simulink model "CCMMotor"  (R2022b)
settings: Solver=FixedStepDiscrete  StartTime=0.0  StopTime=10.0  FixedStep=0.01  RelTol=1e-3
undefined symbols (not defined in the file or in its folder): DcChlType, HFAChlType, MtrRoutStaEnum, P_Std_ReturnType, VGAChlType
(part 1 of 4)
settings Model: Creator=blue.ge  LastModifiedBy=blue.ge
settings DataIOCC: SaveFormat=StructureWithTime
settings OptimizationCC: DefaultParameterBehavior=Inlined  EnhancedBackFolding=on  PassReuseOutputArgsAs=Individual arguments  ZeroExternalMemoryAtStartup=off  ZeroInternalMemoryAtStartup=off  OptimizeBlockOrder=speed  DifferentSizesBufferReuse=on  OptimizationCustomize=off  EfficientTunableParamExpr=on
settings DebuggingCC: ParameterTunabilityLossMsg=error
settings ModelReferenceCC: ModelReferenceNumInstancesAllowed=Single
settings SFSimCC: MATLABDynamicMemAlloc=off
settings RTWCC: Description=AUTOSAR  SystemTargetFile=autosar.tlc  GenCodeOnly=on  TemplateMakefile=ert_default_tmf
settings CodeAppCC: ForceParamTrailComments=on  IgnoreCustomStorageClasses=off  OperatorAnnotations=on  SimulinkDataObjDesc=on  SFDataObjDesc=on  InsertBlockDesc=on
settings STFCustomTargetCC: TargetLangStandard=C99 (ISO)  MultiwordLength=256  ParMdlRefBuildCompliant=on  CompOptLevelCompliant=on  IncludeMdlTerminateFcn=off  CombineOutputUpdateFcns=on  SuppressErrorStatus=on  ERTCustomFileBanners=on  SupportNonFinite=off  SupportComplex=off  SupportContinuousTime=off  SupportNonInlinedSFcns=off  RemoveResetFunc=on  MATLABClassNameForMDSCustomization=Simulink.SoftwareTarget.ERTCustomization  AutosarCompliant=on  UseToolchainInfoCompliant=on  LookupTableObjectStructAxisOrder=2,1,3,4,...  LUTObjectStructOrderEvenSpacing=Size,Table,Breakpoints  SystemTargetFile=autosar.tlc
settings ERTTargetCC: TargetLangStandard=C99 (ISO)  ConcurrentExecutionCompliant=off  IncludeMdlTerminateFcn=off  SuppressErrorStatus=on  SupportNonFinite=off  SupportComplex=off  ModelStepFunctionPrototypeControlCompliant=off  CPPClassGenCompliant=off  AutosarCompliant=on  LookupTableObjectStructAxisOrder=2,1,3,4,...  LUTObjectStructOrderEvenSpacing=Size,Table,Breakpoints  InlinedParameterPlacement=NonHierarchical  ERTCustomFileTemplate=example_file_process.tlc

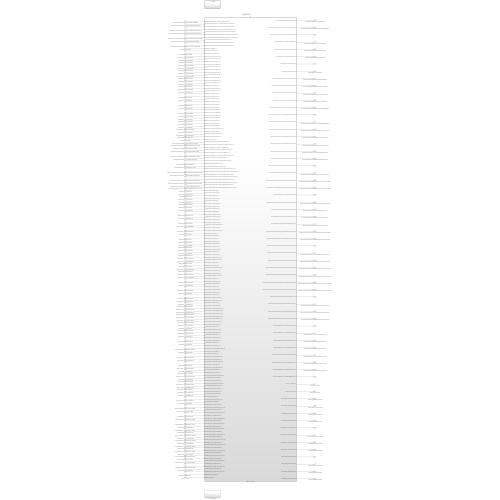
[thumbnail: Simulink's stored preview of the top-level diagram]
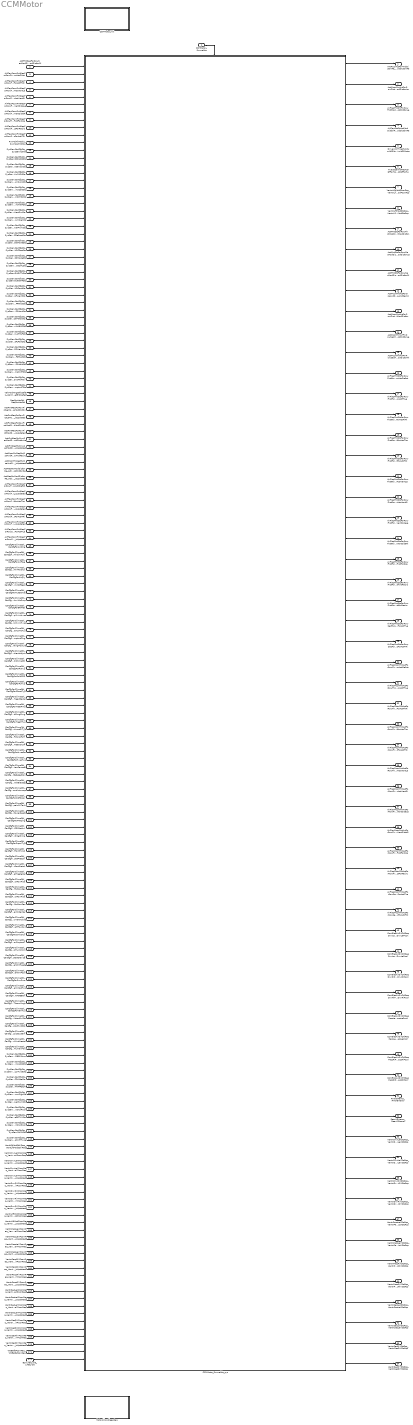
== system CCMMotor ==
  CCMMotor_Runnable : Inport  SampleTime=0.01  OutputFunctionCall=on
  ActrFrntReqForRowSecModDistbnRi_ActrFrntReqForRowSecModDistbnRi : Inport  Port=2  OutMin=0  OutMax=100  OutDataTypeStr=Perc9  PortDimensions=1
  AirFlapPosnFrntReqForRowFirstForActrFlrLe_AirFlapPosnFrntReqForRowFirstForActrFlrLe : Inport  Port=3  OutMin=0  OutMax=100  OutDataTypeStr=Perc9  PortDimensions=1
  AirFlapPosnFrntReqForRowFirstForActrFlrRi_AirFlapPosnFrntReqForRowFirstForActrFlrRi : Inport  Port=4  OutMin=0  OutMax=100  OutDataTypeStr=Perc9  PortDimensions=1
  AirFlapPosnFrntReqForRowFirstForActrVentLe_AirFlapPosnFrntReqForRowFirstForActrVentLe : Inport  Port=5  OutMin=0  OutMax=100  OutDataTypeStr=Perc9  PortDimensions=1
  AirFlapPosnFrntReqForRowFirstForActrVentRi_AirFlapPosnFrntReqForRowFirstForActrVentRi : Inport  Port=6  OutMin=0  OutMax=100  OutDataTypeStr=Perc9  PortDimensions=1
  AirFlapPosnFrntReqForRowFirstForActrVentSideLe_AirFlapPosnFrntReqForRowFirstForActrVentSideLe : Inport  Port=7  OutMin=0  OutMax=100  OutDataTypeStr=Perc9  PortDimensions=1
  AirFlapPosnFrntReqForRowFirstForActrVentSideRi_AirFlapPosnFrntReqForRowFirstForActrVentSideRi : Inport  Port=8  OutMin=0  OutMax=100  OutDataTypeStr=Perc9  PortDimensions=1
  AirFlapPosnFrntReqForRowFirstForOsa_AirFlapPosnFrntReqForRowFirstForOsa : Inport  Port=9  OutMin=0  OutMax=100  OutDataTypeStr=Perc9  PortDimensions=1
  AirFlapPosnFrntReqForRowFirstForRecirc_AirFlapPosnFrntReqForRowFirstForRecirc : Inport  Port=10  OutMin=0  OutMax=100  OutDataTypeStr=Perc9  PortDimensions=1
  AirFlapPosnFrntReqForRowSecForActrTRi_AirFlapPosnFrntReqForRowSecForActrTRi : Inport  Port=11  OutMin=0  OutMax=100  OutDataTypeStr=Perc9  PortDimensions=1
  PwrMdAllMtrSlp_PwrMdAllMtrSlp : Inport  Port=12  OutDataTypeStr=Enum: PwrMdAllMtrSlp  PortDimensions=1
  SysServCarCfgSts_SysServCarID : Inport  Port=13  OutDataTypeStr=Enum: SysServCarID  PortDimensions=1
  SysServCarCfgSts_SysServCCPAQSSts : Inport  Port=14  OutDataTypeStr=UInt8  PortDimensions=1
  SysServCarCfgSts_SysServCCPHEXSensorSts : Inport  Port=15  OutDataTypeStr=UInt8  PortDimensions=1
  SysServCarCfgSts_SysServCCPLin2AFUSts : Inport  Port=16  OutDataTypeStr=Boolean  PortDimensions=1
  SysServCarCfgSts_SysServCCPLin2AIUSts : Inport  Port=17  OutDataTypeStr=UInt8  PortDimensions=1
  SysServCarCfgSts_SysServCCPLin2CDSSts : Inport  Port=18  OutDataTypeStr=Boolean  PortDimensions=1
  SysServCarCfgSts_SysServCCPLin2PMSISts : Inport  Port=19  OutDataTypeStr=Boolean  PortDimensions=1
  SysServCarCfgSts_SysServCCPLin3VGASts : Inport  Port=20  OutDataTypeStr=Boolean  PortDimensions=1
  SysServCarCfgSts_SysServDUAirVentSnrSts : Inport  Port=21  OutDataTypeStr=Boolean  PortDimensions=1
  SysServCarCfgSts_SysServDUBlowerPwmSignSts : Inport  Port=22  OutDataTypeStr=Boolean  PortDimensions=1
  SysServCarCfgSts_SysServDUBlowerPwmSts : Inport  Port=23  OutDataTypeStr=Boolean  PortDimensions=1
  SysServCarCfgSts_SysServDUDCDefrostSts : Inport  Port=24  OutDataTypeStr=UInt8  PortDimensions=1
  SysServCarCfgSts_SysServDUDCModeSts : Inport  Port=25  OutDataTypeStr=UInt8  PortDimensions=1
  SysServCarCfgSts_SysServDUDCRecSts : Inport  Port=26  OutDataTypeStr=UInt8  PortDimensions=1
  SysServCarCfgSts_SysServDUDCSModeSts : Inport  Port=27  OutDataTypeStr=UInt8  PortDimensions=1
  SysServCarCfgSts_SysServDUDCSTLSts : Inport  Port=28  OutDataTypeStr=UInt8  PortDimensions=1
  SysServCarCfgSts_SysServDUDCTLSts : Inport  Port=29  OutDataTypeStr=UInt8  PortDimensions=1
  SysServCarCfgSts_SysServDUDCTRSts : Inport  Port=30  OutDataTypeStr=UInt8  PortDimensions=1
  SysServCarCfgSts_SysServDUFLFootSts : Inport  Port=31  OutDataTypeStr=UInt8  PortDimensions=1
  SysServCarCfgSts_SysServDUFLVentSts : Inport  Port=32  OutDataTypeStr=UInt8  PortDimensions=1
  SysServCarCfgSts_SysServDUFRFootSts : Inport  Port=33  OutDataTypeStr=UInt8  PortDimensions=1
  SysServCarCfgSts_SysServDUFRVentSts : Inport  Port=34  OutDataTypeStr=UInt8  PortDimensions=1
  SysServCarCfgSts_SysServDUIncarMotorSts : Inport  Port=35  OutDataTypeStr=Boolean  PortDimensions=1
  SysServCarCfgSts_SysServDULin1HBMFSts : Inport  Port=36  OutDataTypeStr=Boolean  PortDimensions=1
  SysServCarCfgSts_SysServDULin4HFAFSts : Inport  Port=37  OutDataTypeStr=UInt32  PortDimensions=1
  SysServCarCfgSts_SysServDUSFLFootSts : Inport  Port=38  OutDataTypeStr=UInt8  PortDimensions=1
  SysServCarCfgSts_SysServDUSFLVentSts : Inport  Port=39  OutDataTypeStr=UInt8  PortDimensions=1
  SysServCarCfgSts_SysServDUSFRFootSts : Inport  Port=40  OutDataTypeStr=UInt8  PortDimensions=1
  SysServCarCfgSts_SysServDUSFRVentSts : Inport  Port=41  OutDataTypeStr=UInt8  PortDimensions=1
  SysServCarCfgSts_SysServDUSnsrForFCmptmtTSts : Inport  Port=42  OutDataTypeStr=UInt8  PortDimensions=1
  SysServCarCfgSts_SysServDUSnsrForRair : Inport  Port=43  OutDataTypeStr=UInt8  PortDimensions=1
  SysServCarCfgSts_SysServDUSnsrForRCmptmtTSts : Inport  Port=44  OutDataTypeStr=UInt8  PortDimensions=1
  VehModMngtGlbSafe1_VehModMngtGlbSafe1 : Inport  Port=45  OutDataTypeStr=Bus: VehModMngtGlbSafe1  PortDimensions=1  BusOutputAsStruct=on
  ReqForActrCal_ReqForActrCal : Inport  Port=46  OutDataTypeStr=Boolean  PortDimensions=1
  ActrFrntReqForRowFirstLeModDistbn_ActrFrntReqForRowFirstLeModDistbn : Inport  Port=47  OutMin=0  OutMax=100  OutDataTypeStr=Perc9  PortDimensions=1
  ActrFrntReqForRowFirstLeModDistbn_ActrFrntReqForRowFirstLeModDistbn_IsUpdated : Inport  Port=48  OutDataTypeStr=boolean  PortDimensions=1
  ActrFrntReqForRowFirstModDistbn_ActrFrntReqForRowFirstModDistbn : Inport  Port=49  OutMin=0  OutMax=100  OutDataTypeStr=Perc9  PortDimensions=1
  ActrFrntReqForRowFirstModDistbn_ActrFrntReqForRowFirstModDistbn_IsUpdated : Inport  Port=50  OutDataTypeStr=boolean  PortDimensions=1
  ActrFrntReqForRowSecModDistbnLe_ActrFrntReqForRowSecModDistbnLe : Inport  Port=51  OutMin=0  OutMax=100  OutDataTypeStr=Perc9  PortDimensions=1
  ActrFrntReqForRowSecModDistbnLe_ActrFrntReqForRowSecModDistbnLe_IsUpdated : Inport  Port=52  OutDataTypeStr=boolean  PortDimensions=1
  ActrPosnFrnReqForOsaAndRecirc_ActrPosnFrnReqForOsaAndRecirc : Inport  Port=53  OutMin=0  OutMax=100  OutDataTypeStr=Perc9  PortDimensions=1
  ActrPosnFrnReqForOsaAndRecirc_ActrPosnFrnReqForOsaAndRecirc_IsUpdated : Inport  Port=54  OutDataTypeStr=boolean  PortDimensions=1
  ActrReqForModDistbnRe_ActrReqForModDistbnRe : Inport  Port=55  OutMin=0  OutMax=100  OutDataTypeStr=Perc9  PortDimensions=1
  ActrReqForModDistbnRe_ActrReqForModDistbnRe_IsUpdated : Inport  Port=56  OutDataTypeStr=boolean  PortDimensions=1
  AirFlapPosnFrntReqForRowFirstForActrDefrst_AirFlapPosnFrntReqForRowFirstForActrDefrst : Inport  Port=57  OutMin=0  OutMax=100  OutDataTypeStr=Perc9  PortDimensions=1
  AirFlapPosnFrntReqForRowFirstForActrDefrst_AirFlapPosnFrntReqForRowFirstForActrDefrst_IsUpdated : Inport  Port=58  OutDataTypeStr=boolean  PortDimensions=1
  AirFlapPosnFrntReqForRowFirstForActrTLe_AirFlapPosnFrntReqForRowFirstForActrTLe : Inport  Port=59  OutMin=0  OutMax=100  OutDataTypeStr=Perc9  PortDimensions=1
  AirFlapPosnFrntReqForRowFirstForActrTLe_AirFlapPosnFrntReqForRowFirstForActrTLe_IsUpdated : Inport  Port=60  OutDataTypeStr=boolean  PortDimensions=1
  AirFlapPosnFrntReqForRowFirstForActrTRi_AirFlapPosnFrntReqForRowFirstForActrTRi : Inport  Port=61  OutMin=0  OutMax=100  OutDataTypeStr=Perc9  PortDimensions=1
  AirFlapPosnFrntReqForRowFirstForActrTRi_AirFlapPosnFrntReqForRowFirstForActrTRi_IsUpdated : Inport  Port=62  OutDataTypeStr=boolean  PortDimensions=1
  AirFlapPosnFrntReqForRowSecForActrTLe_AirFlapPosnFrntReqForRowSecForActrTLe : Inport  Port=63  OutMin=0  OutMax=100  OutDataTypeStr=Perc9  PortDimensions=1
  AirFlapPosnFrntReqForRowSecForActrTLe_AirFlapPosnFrntReqForRowSecForActrTLe_IsUpdated : Inport  Port=64  OutDataTypeStr=boolean  PortDimensions=1
  CarCfgForClimaCtrl_CarCfgForAirClng : Inport  Port=65  OutDataTypeStr=Enum: CarCfgForAirClng  PortDimensions=1
  CarCfgForClimaCtrl_CarCfgForAirClnrAdv : Inport  Port=66  OutDataTypeStr=UInt8  PortDimensions=1
  CarCfgForClimaCtrl_CarCfgForAirFrag : Inport  Port=67  OutDataTypeStr=Enum: CarCfgForAirFrag  PortDimensions=1
  CarCfgForClimaCtrl_CarCfgForAirMtrlSnsr : Inport  Port=68  OutDataTypeStr=Enum: CarCfgForAirMtrlSnsrGly  PortDimensions=1
  CarCfgForClimaCtrl_CarCfgForAirQly : Inport  Port=69  OutDataTypeStr=Enum: CarCfgForAirQlyGly  PortDimensions=1
  CarCfgForClimaCtrl_CarCfgForAmntOfSeat : Inport  Port=70  OutDataTypeStr=Enum: CarCfgForAmntOfSeatTyp3  PortDimensions=1
  CarCfgForClimaCtrl_CarCfgForAutDemst : Inport  Port=71  OutDataTypeStr=UInt8  PortDimensions=1
  CarCfgForClimaCtrl_CarCfgForAuxWtrPmp : Inport  Port=72  OutDataTypeStr=Enum: CarCfgForAuxWtrPmp  PortDimensions=1
  CarCfgForClimaCtrl_CarCfgForBRAND : Inport  Port=73  OutDataTypeStr=UInt8  PortDimensions=1
  CarCfgForClimaCtrl_CarCfgForBypVlvInHvac : Inport  Port=74  OutDataTypeStr=Enum: CarCfgForBypVlvInHvac2  PortDimensions=1
  CarCfgForClimaCtrl_CarCfgForClsVlvInHvac : Inport  Port=75  OutDataTypeStr=Enum: CarCfgForClsVlvInHvac  PortDimensions=1
  CarCfgForClimaCtrl_CarCfgForCmprAcTyp : Inport  Port=76  OutDataTypeStr=UInt8  PortDimensions=1
  CarCfgForClimaCtrl_CarCfgForCmptmtClng : Inport  Port=77  OutDataTypeStr=Enum: CarCfgForCmptmtClng  PortDimensions=1
  CarCfgForClimaCtrl_CarCfgForElectricLiftingModule : Inport  Port=78  OutDataTypeStr=UInt8  PortDimensions=1
  CarCfgForClimaCtrl_CarCfgForElecVentAdjmt : Inport  Port=79  OutDataTypeStr=Enum: CarCfgForElecVentAdjmt  PortDimensions=1
  CarCfgForClimaCtrl_CarCfgForExtVentnClimaCtrl : Inport  Port=80  OutDataTypeStr=Enum: CarCfgForExtVentnClimaCtrl  PortDimensions=1
  CarCfgForClimaCtrl_CarCfgForFlcTyp : Inport  Port=81  OutDataTypeStr=Enum: CarCfgForFlcTyp  PortDimensions=1
  CarCfgForClimaCtrl_CarCfgForFoldCSD : Inport  Port=82  OutDataTypeStr=Enum: CarCfgForFoldCSD  PortDimensions=1
  CarCfgForClimaCtrl_CarCfgForFuTyp : Inport  Port=83  OutDataTypeStr=Enum: CarCfgForFuTyp  PortDimensions=1
  CarCfgForClimaCtrl_CarCfgForGlassTyp : Inport  Port=84  OutDataTypeStr=Enum: CarCfgForGlassTyp  PortDimensions=1
  CarCfgForClimaCtrl_CarCfgForHeadRadar : Inport  Port=85  OutDataTypeStr=Enum: HeadRadar  PortDimensions=1
  CarCfgForClimaCtrl_CarCfgForHeatPmp : Inport  Port=86  OutDataTypeStr=Enum: CarCfgForHeatPmpTyp  PortDimensions=1
  CarCfgForClimaCtrl_CarCfgForHeatrDurgDrvg : Inport  Port=87  OutDataTypeStr=Enum: CarCfgForHeatrDurgDrvg  PortDimensions=1
  CarCfgForClimaCtrl_CarCfgForHeatrTyp : Inport  Port=88  OutDataTypeStr=UInt8  PortDimensions=1
  CarCfgForClimaCtrl_CarCfgForHvacFilTyp : Inport  Port=89  OutDataTypeStr=Enum: CarCfgForHvacFilTypGly  PortDimensions=1
  CarCfgForClimaCtrl_CarCfgForHvacFlrSnsrForT : Inport  Port=90  OutDataTypeStr=Enum: CarCfgForHvacFlrSnsrForT  PortDimensions=1
  CarCfgForClimaCtrl_CarCfgForHvacHeatrSnsrT : Inport  Port=91  OutDataTypeStr=Enum: CarCfgForHvacHeatrSnsrT  PortDimensions=1
  CarCfgForClimaCtrl_CarCfgForHvacRe : Inport  Port=92  OutDataTypeStr=UInt8  PortDimensions=1
  CarCfgForClimaCtrl_CarCfgForHvacTyp : Inport  Port=93  OutDataTypeStr=UInt8  PortDimensions=1
  CarCfgForClimaCtrl_CarCfgForInteriorViewCamera : Inport  Port=94  OutDataTypeStr=Enum: CarCfgForInteriorViewCamera  PortDimensions=1
  CarCfgForClimaCtrl_CarCfgForLifeDetection : Inport  Port=95  OutDataTypeStr=Enum: LifeDetection  PortDimensions=1
  CarCfgForClimaCtrl_CarCfgForLoVoltElecCfg : Inport  Port=96  OutDataTypeStr=Enum: CarCfgForLoVoltElecCfg  PortDimensions=1
  CarCfgForClimaCtrl_CarCfgForNavSysandIntnmap : Inport  Port=97  OutDataTypeStr=Enum: CarCfgForNavSysandIntnmap  PortDimensions=1
  CarCfgForClimaCtrl_CarCfgForNrOfDoor : Inport  Port=98  OutDataTypeStr=Enum: CarCfgForNrOfDoor2  PortDimensions=1
  CarCfgForClimaCtrl_CarCfgForPipeInFuTank : Inport  Port=99  OutDataTypeStr=Enum: CarCfgForPipeInFuTank  PortDimensions=1
  CarCfgForClimaCtrl_CarCfgForPowerSeats : Inport  Port=100  OutDataTypeStr=Enum: CarCfgForPowerSeats  PortDimensions=1
  CarCfgForClimaCtrl_CarCfgForPreClng : Inport  Port=101  OutDataTypeStr=Enum: CarCfgForPreClng  PortDimensions=1
  CarCfgForClimaCtrl_CarCfgForPrkgCDSNotAvl : Inport  Port=102  OutDataTypeStr=Enum: CarCfgForPrkgCDSNotAvl  PortDimensions=1
  CarCfgForClimaCtrl_CarCfgForPrkgClima : Inport  Port=103  OutDataTypeStr=Enum: CarCfgForPrkgClima  PortDimensions=1
  CarCfgForClimaCtrl_CarCfgForPrpsnTyp : Inport  Port=104  OutDataTypeStr=Enum: CarCfgForPrpsnTypGly  PortDimensions=1
  CarCfgForClimaCtrl_CarCfgForPTCInFrontHVAC : Inport  Port=105  OutDataTypeStr=UInt8  PortDimensions=1
  CarCfgForClimaCtrl_CarCfgForRemEngStrtPreCdn : Inport  Port=106  OutDataTypeStr=Enum: CarCfgForRemEngStrtPreCdn  PortDimensions=1
  CarCfgForClimaCtrl_CarCfgForRoofHatch : Inport  Port=107  OutDataTypeStr=Enum: CarCfgForRoofHatch  PortDimensions=1
  CarCfgForClimaCtrl_CarCfgForSeatHeatgCal : Inport  Port=108  OutDataTypeStr=Enum: CarCfgForSeatHeatgCal1  PortDimensions=1
  CarCfgForClimaCtrl_CarCfgForSeatHeatgForRowFirst : Inport  Port=109  OutDataTypeStr=Enum: CarCfgForSeatHeatgForRowFirst  PortDimensions=1
  CarCfgForClimaCtrl_CarCfgForSeatHeatgForRowSec : Inport  Port=110  OutDataTypeStr=Enum: CarCfgForSeatHeatgForRowSec  PortDimensions=1
  CarCfgForClimaCtrl_CarCfgForSeatVentnForRowFirst : Inport  Port=111  OutDataTypeStr=Enum: CarCfgForSeatVentnForRowFirst1  PortDimensions=1
  CarCfgForClimaCtrl_CarCfgForSeatVentnForRowSec : Inport  Port=112  OutDataTypeStr=Enum: CarCfgForSeatVentnForRowSecGly  PortDimensions=1
  CarCfgForClimaCtrl_CarCfgForSecRowVentnCenter : Inport  Port=113  OutDataTypeStr=Enum: CarCfgForSecRowVentnCenter  PortDimensions=1
  CarCfgForClimaCtrl_CarCfgForSecRowVentnSide : Inport  Port=114  OutDataTypeStr=Enum: CarCfgForSecRowVentnSide  PortDimensions=1
  CarCfgForClimaCtrl_CarCfgForSmallFlowCyc : Inport  Port=115  OutDataTypeStr=Enum: CarCfgForSmallFlowCyc  PortDimensions=1
  CarCfgForClimaCtrl_CarCfgForSmkSnsr : Inport  Port=116  OutDataTypeStr=Enum: CarCfgForSmkSnsr  PortDimensions=1
  CarCfgForClimaCtrl_CarCfgForSnsrForCmptmtT : Inport  Port=117  OutDataTypeStr=Enum: CarCfgForSnsrForCmptmtT  PortDimensions=1
  CarCfgForClimaCtrl_CarCfgForSnsrHumPrsnt : Inport  Port=118  OutDataTypeStr=Enum: CarCfgForSnsrHumPrsnt  PortDimensions=1
  CarCfgForClimaCtrl_CarCfgForStartDevice : Inport  Port=119  OutDataTypeStr=Enum: StartDevice  PortDimensions=1
  CarCfgForClimaCtrl_CarCfgForSteerWhlHeatg : Inport  Port=120  OutDataTypeStr=UInt8  PortDimensions=1
  CarCfgForClimaCtrl_CarCfgForSteerWhlPosn : Inport  Port=121  OutDataTypeStr=Enum: CarCfgForSteerWhlPosn  PortDimensions=1
  CarCfgForClimaCtrl_CarCfgForSunSnsr : Inport  Port=122  OutDataTypeStr=Enum: CarCfgForSunSnsr  PortDimensions=1
  CarCfgForClimaCtrl_CarCfgForTelmNodTyp : Inport  Port=123  OutDataTypeStr=Enum: CarCfgForTelmNodTyp  PortDimensions=1
  CarCfgForClimaCtrl_CarCfgForTEMPEREDSTORAGEBOX : Inport  Port=124  OutDataTypeStr=UInt8  PortDimensions=1
  CarCfgForClimaCtrl_CarCfgForThermMngt : Inport  Port=125  OutDataTypeStr=Enum: CarCfgForThermMngt  PortDimensions=1
  CarCfgForClimaCtrl_CarCfgForVehTyp : Inport  Port=126  OutDataTypeStr=Enum: CarCfgForVehTyp3  PortDimensions=1
  CarCfgForClimaCtrl_CarCfgForVentLiftMotorExiSts : Inport  Port=127  OutDataTypeStr=UInt32  PortDimensions=1
  CarCfgForClimaCtrl_CarCfgForVgaMotorExistSts : Inport  Port=128  OutDataTypeStr=CarCfgForVgaMotorExistSts  PortDimensions=1
  CarCfgForClimaCtrl_CarCfgForWadingAssistSystem : Inport  Port=129  OutDataTypeStr=UInt8  PortDimensions=1
  CarCfgForClimaCtrl_CarCfgForWindHeatd : Inport  Port=130  OutDataTypeStr=Enum: CarCfgForWindHeatd  PortDimensions=1
  CarCfgForClimaCtrl_CarCfgWindFollowHumanMov : Inport  Port=131  OutDataTypeStr=UInt8  PortDimensions=1
  SysServCarCfgSts_SysServCCPBGMNum : Inport  Port=132  OutDataTypeStr=UInt8  PortDimensions=1
  SysServCarCfgSts_SysServCCPLin2AQSSts : Inport  Port=133  OutDataTypeStr=Boolean  PortDimensions=1
  SysServCarCfgSts_SysServDUBlowerPwmSignTypeSts : Inport  Port=134  OutDataTypeStr=UInt8  PortDimensions=1
  SysServCarCfgSts_SysServDUDCFLMdeSts : Inport  Port=135  OutDataTypeStr=UInt8  PortDimensions=1
  SysServCarCfgSts_SysServDULin4HFAFStsH : Inport  Port=136  OutDataTypeStr=UInt32  PortDimensions=1
  SysServCarCfgSts_SysServDUReBlowerPwmSignSts : Inport  Port=137  OutDataTypeStr=UInt8  PortDimensions=1
  SysServCarCfgSts_SysServDUReBlowerPwmSts : Inport  Port=138  OutDataTypeStr=UInt8  PortDimensions=1
  SysServCarCfgSts_SysServFrntHEXSnsrPosn : Inport  Port=139  OutDataTypeStr=UInt8  PortDimensions=1
  SysServCarCfgSts_SysServLowSideRETXVSts : Inport  Port=140  OutDataTypeStr=UInt8  PortDimensions=1
  SysServCarCfgSts_SysServReHEXSnsrPosn : Inport  Port=141  OutDataTypeStr=UInt8  PortDimensions=1
  SysServCarCfgSts_SysServRHFASts : Inport  Port=142  OutDataTypeStr=UInt8  PortDimensions=1
  SysServCarCfgSts_SysServSnsrForFCmptmtTType : Inport  Port=143  OutDataTypeStr=UInt8  PortDimensions=1
  VentLiftModCaliReq_VentLiftModCaliReq : Inport  Port=144  OutDataTypeStr=UInt8  PortDimensions=1
  VentnDrvrLeXPsonReq_VentnDrvrLeXPsonReq : Inport  Port=145  OutDataTypeStr=UInt16  PortDimensions=1
  VentnDrvrLeXPsonReq_VentnDrvrLeXPsonReq_IsUpdated : Inport  Port=146  OutDataTypeStr=boolean  PortDimensions=1
  VentnDrvrLeYPsonReq_VentnDrvrLeYPsonReq : Inport  Port=147  OutDataTypeStr=UInt16  PortDimensions=1
  VentnDrvrLeYPsonReq_VentnDrvrLeYPsonReq_IsUpdated : Inport  Port=148  OutDataTypeStr=boolean  PortDimensions=1
  VentnDrvrRiXPsonReq_VentnDrvrRiXPsonReq : Inport  Port=149  OutDataTypeStr=UInt16  PortDimensions=1
  VentnDrvrRiXPsonReq_VentnDrvrRiXPsonReq_IsUpdated : Inport  Port=150  OutDataTypeStr=boolean  PortDimensions=1
  VentnDrvrRiYPsonReq_VentnDrvrRiYPsonReq : Inport  Port=151  OutDataTypeStr=UInt16  PortDimensions=1
  VentnDrvrRiYPsonReq_VentnDrvrRiYPsonReq_IsUpdated : Inport  Port=152  OutDataTypeStr=boolean  PortDimensions=1
  VentnLiftModPosnReq_VentnLiftModPosnReq : Inport  Port=153  OutDataTypeStr=UInt8  PortDimensions=1
  VentnLiftModPosnReq_VentnLiftModPosnReq_IsUpdated : Inport  Port=154  OutDataTypeStr=boolean  PortDimensions=1
  VentnPassLeXPsonReq_VentnPassLeXPsonReq : Inport  Port=155  OutDataTypeStr=UInt16  PortDimensions=1
  VentnPassLeXPsonReq_VentnPassLeXPsonReq_IsUpdated : Inport  Port=156  OutDataTypeStr=boolean  PortDimensions=1
  VentnPassLeYPsonReq_VentnPassLeYPsonReq : Inport  Port=157  OutDataTypeStr=UInt16  PortDimensions=1
  VentnPassLeYPsonReq_VentnPassLeYPsonReq_IsUpdated : Inport  Port=158  OutDataTypeStr=boolean  PortDimensions=1
  VentnPassRiXPsonReq_VentnPassRiXPsonReq : Inport  Port=159  OutDataTypeStr=UInt16  PortDimensions=1
  VentnPassRiXPsonReq_VentnPassRiXPsonReq_IsUpdated : Inport  Port=160  OutDataTypeStr=boolean  PortDimensions=1
  VentnPassRiYPsonReq_VentnPassRiYPsonReq : Inport  Port=161  OutDataTypeStr=UInt16  PortDimensions=1
  VentnPassRiYPsonReq_VentnPassRiYPsonReq_IsUpdated : Inport  Port=162  OutDataTypeStr=boolean  PortDimensions=1
  VentnSecLeXPosnReq_VentnSecLeXPosnReq : Inport  Port=163  OutDataTypeStr=UInt16  PortDimensions=1
  VentnSecLeXPosnReq_VentnSecLeXPosnReq_IsUpdated : Inport  Port=164  OutDataTypeStr=boolean  PortDimensions=1
  VentnSecLeYPosnReq_VentnSecLeYPosnReq : Inport  Port=165  OutDataTypeStr=UInt16  PortDimensions=1
  VentnSecLeYPosnReq_VentnSecLeYPosnReq_IsUpdated : Inport  Port=166  OutDataTypeStr=boolean  PortDimensions=1
  VentnSecRiXPosnReq_VentnSecRiXPosnReq : Inport  Port=167  OutDataTypeStr=UInt16  PortDimensions=1
  VentnSecRiXPosnReq_VentnSecRiXPosnReq_IsUpdated : Inport  Port=168  OutDataTypeStr=boolean  PortDimensions=1
  VentnSecRiYPosnReq_VentnSecRiYPosnReq : Inport  Port=169  OutDataTypeStr=UInt16  PortDimensions=1
  VentnSecRiYPosnReq_VentnSecRiYPosnReq_IsUpdated : Inport  Port=170  OutDataTypeStr=boolean  PortDimensions=1
  WtrBafePsonReq_WtrBafePsonReq : Inport  Port=171  OutDataTypeStr=UInt16  PortDimensions=1
  BswLifeCycle_LifeCycle : Inport  Port=172  OutDataTypeStr=Enum: CCMLifeCycleModeGroup  PortDimensions=1
  CCMMotor_Init : Subsystem
  CCMMotor_Runnable_sys : Subsystem
  MotorAllowSleepServ : Subsystem
  ActrPosnActForModDistbnRe_ActrPosnActForModDistbnRe : Outport  OutMin=0  OutMax=100  OutDataTypeStr=Perc9  PortDimensions=1
  ActrPosnFrntActForRowFirstModDistbnLe_ActrPosnFrntActForRowFirstModDistbnLe : Outport  Port=2  OutMin=0  OutMax=100  OutDataTypeStr=Perc9  PortDimensions=1
  AirFlapFrntStsForRowFirstModDistbnLe_AirFlapFrntStsForRowFirstModDistbnLe : Outport  Port=3  OutDataTypeStr=Enum: HvacActrSts  PortDimensions=1
  AirFlapStsForActModDistbnRe_AirFlapStsForActModDistbnRe : Outport  Port=4  OutDataTypeStr=Enum: HvacActrSts  PortDimensions=1
  DriverVolThresholdLimitOfMotor_DriverVolThresholdLimitOfMotor : Outport  Port=5  OutDataTypeStr=UInt16  PortDimensions=1
  HvacOsaAndRecActrQfForHp_HvacOsaAndRecActrQfForHp : Outport  Port=6  OutDataTypeStr=Enum: Err1  PortDimensions=1
  VentnLiftModPosnRsp_VentnLiftModPosnRsp : Outport  Port=7  OutDataTypeStr=UInt8  PortDimensions=1
  VentnLiftModStsRsp_VentnLiftModStsRsp : Outport  Port=8  OutDataTypeStr=Enum: VentnStsRsp  PortDimensions=1
  ActrFrntStsForRowFirstModDistbn_ActrFrntStsForRowFirstModDistbn : Outport  Port=9  OutDataTypeStr=Enum: HvacActrSts  PortDimensions=1
  ActrFrntStsForRowSecModDistbnLe_ActrFrntStsForRowSecModDistbnLe : Outport  Port=10  OutDataTypeStr=Enum: HvacActrSts  PortDimensions=1
  ActrFrntStsForRowSecModDistbnRi_ActrFrntStsForRowSecModDistbnRi : Outport  Port=11  OutDataTypeStr=Enum: HvacActrSts  PortDimensions=1
  ActrPosnFrntActForOsaAndRecirc_ActrPosnFrntActForOsaAndRecirc : Outport  Port=12  OutMin=0  OutMax=100  OutDataTypeStr=Perc9  PortDimensions=1
  ActrPosnFrntActForRowFirstModDistbn_ActrPosnFrntActForRowFirstModDistbn : Outport  Port=13  OutMin=0  OutMax=100  OutDataTypeStr=Perc9  PortDimensions=1
  ActrPosnFrntActForRowSecModDistbnLe_ActrPosnFrntActForRowSecModDistbnLe : Outport  Port=14  OutMin=0  OutMax=100  OutDataTypeStr=Perc9  PortDimensions=1
  ActrPosnFrntActForRowSecModDistbnRi_ActrPosnFrntActForRowSecModDistbnRi : Outport  Port=15  OutMin=0  OutMax=100  OutDataTypeStr=Perc9  PortDimensions=1
  AirFlapFrntStsForRowFirstForActrDefrst_AirFlapFrntStsForRowFirstForActrDefrst : Outport  Port=16  OutDataTypeStr=Enum: HvacActrSts  PortDimensions=1
  AirFlapFrntStsForRowFirstForActrFlrLe_AirFlapFrntStsForRowFirstForActrFlrLe : Outport  Port=17  OutDataTypeStr=Enum: HvacActrSts  PortDimensions=1
  AirFlapFrntStsForRowFirstForActrFlrRi_AirFlapFrntStsForRowFirstForActrFlrRi : Outport  Port=18  OutDataTypeStr=Enum: HvacActrSts  PortDimensions=1
  AirFlapFrntStsForRowFirstForActrTLe_AirFlapFrntStsForRowFirstForActrTLe : Outport  Port=19  OutDataTypeStr=Enum: HvacActrSts  PortDimensions=1
  AirFlapFrntStsForRowFirstForActrTRi_AirFlapFrntStsForRowFirstForActrTRi : Outport  Port=20  OutDataTypeStr=Enum: HvacActrSts  PortDimensions=1
  AirFlapFrntStsForRowFirstForActrVentLe_AirFlapFrntStsForRowFirstForActrVentLe : Outport  Port=21  OutDataTypeStr=Enum: HvacActrSts  PortDimensions=1
  AirFlapFrntStsForRowFirstForActrVentRi_AirFlapFrntStsForRowFirstForActrVentRi : Outport  Port=22  OutDataTypeStr=Enum: HvacActrSts  PortDimensions=1
  AirFlapFrntStsForRowFirstForActrVentSideLe_AirFlapFrntStsForRowFirstForActrVentSideLe : Outport  Port=23  OutDataTypeStr=Enum: HvacActrSts  PortDimensions=1
  AirFlapFrntStsForRowFirstForActrVentSideRi_AirFlapFrntStsForRowFirstForActrVentSideRi : Outport  Port=24  OutDataTypeStr=Enum: HvacActrSts  PortDimensions=1
  AirFlapFrntStsForRowFirstForOsa_AirFlapFrntStsForRowFirstForOsa : Outport  Port=25  OutDataTypeStr=Enum: HvacActrSts  PortDimensions=1
  AirFlapFrntStsForRowFirstForOsaAndRecirc_AirFlapFrntStsForRowFirstForOsaAndRecirc : Outport  Port=26  OutDataTypeStr=Enum: HvacActrSts  PortDimensions=1
  AirFlapFrntStsForRowFirstForRecirc_AirFlapFrntStsForRowFirstForRecirc : Outport  Port=27  OutDataTypeStr=Enum: HvacActrSts  PortDimensions=1
  AirFlapFrntStsForRowSecForActrTLe_AirFlapFrntStsForRowSecForActrTLe : Outport  Port=28  OutDataTypeStr=Enum: HvacActrSts  PortDimensions=1
  AirFlapFrntStsForRowSecForActrTRi_AirFlapFrntStsForRowSecForActrTRi : Outport  Port=29  OutDataTypeStr=Enum: HvacActrSts  PortDimensions=1
  AirFlapPosnFrntActForRowFirstForActrDefrst_AirFlapPosnFrntActForRowFirstForActrDefrst : Outport  Port=30  OutMin=0  OutMax=100  OutDataTypeStr=Perc9  PortDimensions=1
  AirFlapPosnFrntActForRowFirstForActrFlrLe_AirFlapPosnFrntActForRowFirstForActrFlrLe : Outport  Port=31  OutMin=0  OutMax=100  OutDataTypeStr=Perc9  PortDimensions=1
  AirFlapPosnFrntActForRowFirstForActrFlrRi_AirFlapPosnFrntActForRowFirstForActrFlrRi : Outport  Port=32  OutMin=0  OutMax=100  OutDataTypeStr=Perc9  PortDimensions=1
  AirFlapPosnFrntActForRowFirstForActrTLe_AirFlapPosnFrntActForRowFirstForActrTLe : Outport  Port=33  OutMin=0  OutMax=100  OutDataTypeStr=Perc9  PortDimensions=1
  AirFlapPosnFrntActForRowFirstForActrTRi_AirFlapPosnFrntActForRowFirstForActrTRi : Outport  Port=34  OutMin=0  OutMax=100  OutDataTypeStr=Perc9  PortDimensions=1
  AirFlapPosnFrntActForRowFirstForActrVentLe_AirFlapPosnFrntActForRowFirstForActrVentLe : Outport  Port=35  OutMin=0  OutMax=100  OutDataTypeStr=Perc9  PortDimensions=1
  AirFlapPosnFrntActForRowFirstForActrVentRi_AirFlapPosnFrntActForRowFirstForActrVentRi : Outport  Port=36  OutMin=0  OutMax=100  OutDataTypeStr=Perc9  PortDimensions=1
  AirFlapPosnFrntActForRowFirstForActrVentSideLe_AirFlapPosnFrntActForRowFirstForActrVentSideLe : Outport  Port=37  OutMin=0  OutMax=100  OutDataTypeStr=Perc9  PortDimensions=1
  AirFlapPosnFrntActForRowFirstForActrVentSideRi_AirFlapPosnFrntActForRowFirstForActrVentSideRi : Outport  Port=38  OutMin=0  OutMax=100  OutDataTypeStr=Perc9  PortDimensions=1
  AirFlapPosnFrntActForRowFirstForOsa_AirFlapPosnFrntActForRowFirstForOsa : Outport  Port=39  OutMin=0  OutMax=100  OutDataTypeStr=Perc9  PortDimensions=1
  AirFlapPosnFrntActForRowFirstForRecirc_AirFlapPosnFrntActForRowFirstForRecirc : Outport  Port=40  OutMin=0  OutMax=100  OutDataTypeStr=Perc9  PortDimensions=1
  AirFlapPosnFrntActForRowSecForActrTLe_AirFlapPosnFrntActForRowSecForActrTLe : Outport  Port=41  OutMin=0  OutMax=100  OutDataTypeStr=Perc9  PortDimensions=1
  AirFlapPosnFrntActForRowSecForActrTRi_AirFlapPosnFrntActForRowSecForActrTRi : Outport  Port=42  OutMin=0  OutMax=100  OutDataTypeStr=Perc9  PortDimensions=1
  CcmElecAirDirCrtlReqDrvrLePosX_CcmElecAirDirCrtlReqDrvrLePosX : Outport  Port=43  OutDataTypeStr=UInt8  PortDimensions=1
  CcmElecAirDirCrtlReqDrvrLePosY_CcmElecAirDirCrtlReqDrvrLePosY : Outport  Port=44  OutDataTypeStr=UInt8  PortDimensions=1
  CcmElecAirDirCrtlReqDrvrRiPosX_CcmElecAirDirCrtlReqDrvrRiPosX : Outport  Port=45  OutDataTypeStr=UInt8  PortDimensions=1
  CcmElecAirDirCrtlReqDrvrRiPosY_CcmElecAirDirCrtlReqDrvrRiPosY : Outport  Port=46  OutDataTypeStr=UInt8  PortDimensions=1
  CcmElecAirDirCrtlReqPassLePosX_CcmElecAirDirCrtlReqPassLePosX : Outport  Port=47  OutDataTypeStr=UInt8  PortDimensions=1
  CcmElecAirDirCrtlReqPassLePosY_CcmElecAirDirCrtlReqPassLePosY : Outport  Port=48  OutDataTypeStr=UInt8  PortDimensions=1
  CcmElecAirDirCrtlReqPassRiPosX_CcmElecAirDirCrtlReqPassRiPosX : Outport  Port=49  OutDataTypeStr=UInt8  PortDimensions=1
  CcmElecAirDirCrtlReqPassRiPosY_CcmElecAirDirCrtlReqPassRiPosY : Outport  Port=50  OutDataTypeStr=UInt8  PortDimensions=1
  FrntVGAerror_FrntVGAerror : Outport  Port=51  OutDataTypeStr=Boolean  PortDimensions=1
  RearVGAerror_RearVGAerror : Outport  Port=52  OutDataTypeStr=Boolean  PortDimensions=1
  VentnDrvrLeXStsRsp_VentnDrvrLeXStsRsp : Outport  Port=53  OutDataTypeStr=Enum: VentnStsRsp  PortDimensions=1
  VentnDrvrLeYStsRsp_VentnDrvrLeYStsRsp : Outport  Port=54  OutDataTypeStr=Enum: VentnStsRsp  PortDimensions=1
  VentnDrvrRiXStsRsp_VentnDrvrRiXStsRsp : Outport  Port=55  OutDataTypeStr=Enum: VentnStsRsp  PortDimensions=1
  VentnDrvrRiYStsRsp_VentnDrvrRiYStsRsp : Outport  Port=56  OutDataTypeStr=Enum: VentnStsRsp  PortDimensions=1
  VentnPassLeXStsRsp_VentnPassLeXStsRsp : Outport  Port=57  OutDataTypeStr=Enum: VentnStsRsp  PortDimensions=1
  VentnPassLeYStsRsp_VentnPassLeYStsRsp : Outport  Port=58  OutDataTypeStr=Enum: VentnStsRsp  PortDimensions=1
  VentnPassRiXStsRsp_VentnPassRiXStsRsp : Outport  Port=59  OutDataTypeStr=Enum: VentnStsRsp  PortDimensions=1
  VentnPassRiYStsRsp_VentnPassRiYStsRsp : Outport  Port=60  OutDataTypeStr=Enum: VentnStsRsp  PortDimensions=1
  VentnSecLeXStsRsp_VentnSecLeXStsRsp : Outport  Port=61  OutDataTypeStr=Enum: VentnStsRsp  PortDimensions=1
  VentnSecLeYStsRsp_VentnSecLeYStsRsp : Outport  Port=62  OutDataTypeStr=Enum: VentnStsRsp  PortDimensions=1
  VentnSecRiXStsRsp_VentnSecRiXStsRsp : Outport  Port=63  OutDataTypeStr=Enum: VentnStsRsp  PortDimensions=1
  VentnSecRiYStsRsp_VentnSecRiYStsRsp : Outport  Port=64  OutDataTypeStr=Enum: VentnStsRsp  PortDimensions=1
  wires:
  ActrFrntReqForRowSecModDistbnRi_ActrFrntReqForRowSecModDistbnRi/1 -> CCMMotor_Runnable_sys/1(ActrFrntReqForRowSecModDistbnRi_ActrFrntReqForRowSecModDistbnRi_read)